simulink model "HvacCtrlrOsaAndRecircFrnt"  (R2022b)
settings: Solver=FixedStepDiscrete  StartTime=0.0  StopTime=10.0  FixedStep=0.2  RelTol=1e-3
settings Model: Creator=blue.ge  LastModifiedBy=blue.ge
settings DataIOCC: SaveFormat=StructureWithTime
settings OptimizationCC: DefaultParameterBehavior=Inlined  EnhancedBackFolding=on  PassReuseOutputArgsAs=Individual arguments  ZeroExternalMemoryAtStartup=off  ZeroInternalMemoryAtStartup=off  OptimizeBlockOrder=speed  DifferentSizesBufferReuse=on  OptimizationCustomize=off  EfficientTunableParamExpr=on
settings DebuggingCC: ParameterTunabilityLossMsg=error
settings ModelReferenceCC: ModelReferenceNumInstancesAllowed=Single
settings SFSimCC: MATLABDynamicMemAlloc=off
settings RTWCC: Description=AUTOSAR  SystemTargetFile=autosar.tlc  GenCodeOnly=on  TemplateMakefile=ert_default_tmf
settings CodeAppCC: ForceParamTrailComments=on  IgnoreCustomStorageClasses=off  OperatorAnnotations=on  SimulinkDataObjDesc=on  SFDataObjDesc=on  InsertBlockDesc=on
settings STFCustomTargetCC: TargetLangStandard=C99 (ISO)  MultiwordLength=256  ParMdlRefBuildCompliant=on  CompOptLevelCompliant=on  IncludeMdlTerminateFcn=off  CombineOutputUpdateFcns=on  SuppressErrorStatus=on  ERTCustomFileBanners=on  SupportNonFinite=off  SupportComplex=off  SupportContinuousTime=off  SupportNonInlinedSFcns=off  RemoveResetFunc=on  MATLABClassNameForMDSCustomization=Simulink.SoftwareTarget.ERTCustomization  AutosarCompliant=on  UseToolchainInfoCompliant=on  LookupTableObjectStructAxisOrder=2,1,3,4,...  LUTObjectStructOrderEvenSpacing=Size,Table,Breakpoints  SystemTargetFile=autosar.tlc
settings ERTTargetCC: TargetLangStandard=C99 (ISO)  ConcurrentExecutionCompliant=off  IncludeMdlTerminateFcn=off  SuppressErrorStatus=on  SupportNonFinite=off  SupportComplex=off  ModelStepFunctionPrototypeControlCompliant=off  CPPClassGenCompliant=off  AutosarCompliant=on  LookupTableObjectStructAxisOrder=2,1,3,4,...  LUTObjectStructOrderEvenSpacing=Size,Table,Breakpoints  InlinedParameterPlacement=NonHierarchical  ERTCustomFileTemplate=example_file_process.tlc

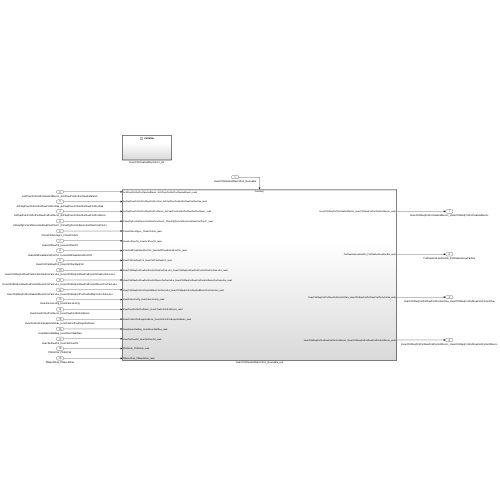
[thumbnail: Simulink's stored preview of the top-level diagram]
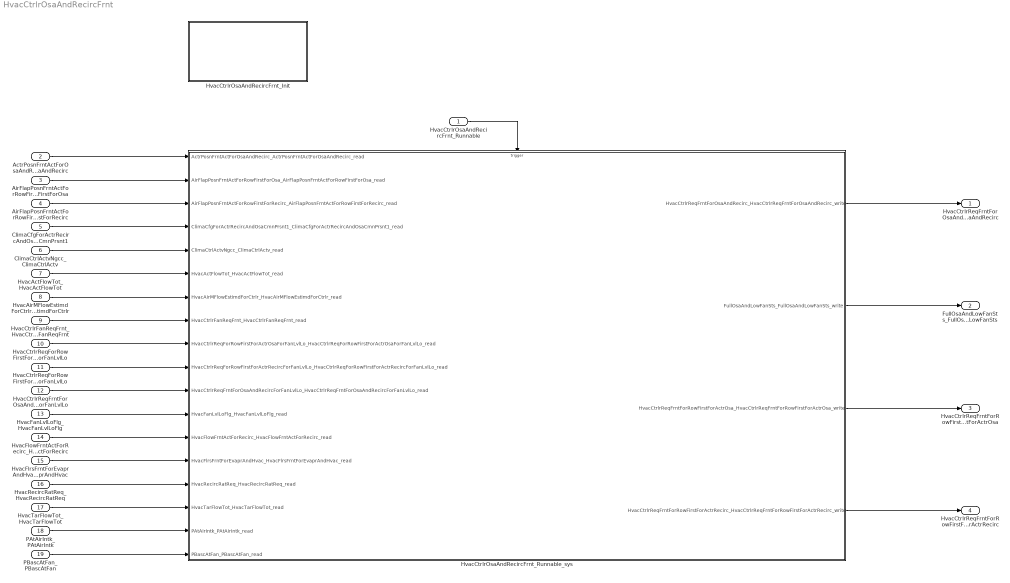
== system HvacCtrlrOsaAndRecircFrnt ==
  HvacCtrlrOsaAndRecircFrnt_Runnable : Inport  SampleTime=0.2  OutputFunctionCall=on
  ActrPosnFrntActForOsaAndRecirc_ActrPosnFrntActForOsaAndRecirc : Inport  Port=2  OutMin=0  OutMax=100  OutDataTypeStr=Perc9  PortDimensions=1
  AirFlapPosnFrntActForRowFirstForOsa_AirFlapPosnFrntActForRowFirstForOsa : Inport  Port=3  OutMin=0  OutMax=100  OutDataTypeStr=Perc9  PortDimensions=1
  AirFlapPosnFrntActForRowFirstForRecirc_AirFlapPosnFrntActForRowFirstForRecirc : Inport  Port=4  OutMin=0  OutMax=100  OutDataTypeStr=Perc9  PortDimensions=1
  ClimaCfgForActrRecircAndOsaCmnPrsnt1_ClimaCfgForActrRecircAndOsaCmnPrsnt1 : Inport  Port=5  OutDataTypeStr=Enum: ClimaCfgForActrRecircAndOsaCmnPrsnt1  PortDimensions=1
  ClimaCtrlActvNgcc_ClimaCtrlActv : Inport  Port=6  OutDataTypeStr=Boolean  PortDimensions=1
  HvacActFlowTot_HvacActFlowTot : Inport  Port=7  OutDataTypeStr=AirFlow30  PortDimensions=1
  HvacAirMFlowEstimdForCtrlr_HvacAirMFlowEstimdForCtrlr : Inport  Port=8  OutDataTypeStr=AirFlow30  PortDimensions=1
  HvacCtrlrFanReqFrnt_HvacCtrlrFanReqFrnt : Inport  Port=9  OutDataTypeStr=FanSpd1  PortDimensions=1
  HvacCtrlrReqForRowFirstForActrOsaForFanLvlLo_HvacCtrlrReqForRowFirstForActrOsaForFanLvlLo : Inport  Port=10  OutDataTypeStr=Perc30  PortDimensions=1
  HvacCtrlrReqForRowFirstForActrRecircForFanLvlLo_HvacCtrlrReqForRowFirstForActrRecircForFanLvlLo : Inport  Port=11  OutDataTypeStr=Perc30  PortDimensions=1
  HvacCtrlrReqFrntForOsaAndRecircForFanLvlLo_HvacCtrlrReqFrntForOsaAndRecircForFanLvlLo : Inport  Port=12  OutDataTypeStr=Perc30  PortDimensions=1
  HvacFanLvlLoFlg_HvacFanLvlLoFlg : Inport  Port=13  OutDataTypeStr=Boolean  PortDimensions=1
  HvacFlowFrntActForRecirc_HvacFlowFrntActForRecirc : Inport  Port=14  OutDataTypeStr=AirFlow30  PortDimensions=1
  HvacFlrsFrntForEvaprAndHvac_HvacFlrsFrntForEvaprAndHvac : Inport  Port=15  OutDataTypeStr=Flrs30  PortDimensions=1
  HvacRecircRatReq_HvacRecircRatReq : Inport  Port=16  OutDataTypeStr=Perc30  PortDimensions=1
  HvacTarFlowTot_HvacTarFlowTot : Inport  Port=17  OutDataTypeStr=AirFlow30  PortDimensions=1
  PAtAirIntk_PAtAirIntk : Inport  Port=18  OutDataTypeStr=AirP31  PortDimensions=1
  PBascAtFan_PBascAtFan : Inport  Port=19  OutDataTypeStr=AirP31  PortDimensions=1
  HvacCtrlrOsaAndRecircFrnt_Init : Subsystem
  HvacCtrlrOsaAndRecircFrnt_Runnable_sys : Subsystem
  HvacCtrlrReqFrntForOsaAndRecirc_HvacCtrlrReqFrntForOsaAndRecirc : Outport  OutDataTypeStr=Perc30  PortDimensions=1
  FullOsaAndLowFanSts_FullOsaAndLowFanSts : Outport  Port=2  OutDataTypeStr=Boolean  PortDimensions=1
  HvacCtrlrReqFrntForRowFirstForActrOsa_HvacCtrlrReqFrntForRowFirstForActrOsa : Outport  Port=3  OutDataTypeStr=Perc30  PortDimensions=1
  HvacCtrlrReqFrntForRowFirstForActrRecirc_HvacCtrlrReqFrntForRowFirstForActrRecirc : Outport  Port=4  OutDataTypeStr=Perc30  PortDimensions=1
  wires:
  ActrPosnFrntActForOsaAndRecirc_ActrPosnFrntActForOsaAndRecirc/1 -> HvacCtrlrOsaAndRecircFrnt_Runnable_sys/1(ActrPosnFrntActForOsaAndRecirc_ActrPosnFrntActForOsaAndRecirc_read)
  AirFlapPosnFrntActForRowFirstForOsa_AirFlapPosnFrntActForRowFirstForOsa/1 -> HvacCtrlrOsaAndRecircFrnt_Runnable_sys/2(AirFlapPosnFrntActForRowFirstForOsa_AirFlapPosnFrntActForRowFirstForOsa_read)
  AirFlapPosnFrntActForRowFirstForRecirc_AirFlapPosnFrntActForRowFirstForRecirc/1 -> HvacCtrlrOsaAndRecircFrnt_Runnable_sys/3(AirFlapPosnFrntActForRowFirstForRecirc_AirFlapPosnFrntActForRowFirstForRecirc_read)
  ClimaCfgForActrRecircAndOsaCmnPrsnt1_ClimaCfgForActrRecircAndOsaCmnPrsnt1/1 -> HvacCtrlrOsaAndRecircFrnt_Runnable_sys/4(ClimaCfgForActrRecircAndOsaCmnPrsnt1_ClimaCfgForActrRecircAndOsaCmnPrsnt1_read)
  ClimaCtrlActvNgcc_ClimaCtrlActv/1 -> HvacCtrlrOsaAndRecircFrnt_Runnable_sys/5(ClimaCtrlActvNgcc_ClimaCtrlActv_read)
  HvacActFlowTot_HvacActFlowTot/1 -> HvacCtrlrOsaAndRecircFrnt_Runnable_sys/6(HvacActFlowTot_HvacActFlowTot_read)
  HvacAirMFlowEstimdForCtrlr_HvacAirMFlowEstimdForCtrlr/1 -> HvacCtrlrOsaAndRecircFrnt_Runnable_sys/7(HvacAirMFlowEstimdForCtrlr_HvacAirMFlowEstimdForCtrlr_read)
  HvacCtrlrFanReqFrnt_HvacCtrlrFanReqFrnt/1 -> HvacCtrlrOsaAndRecircFrnt_Runnable_sys/8(HvacCtrlrFanReqFrnt_HvacCtrlrFanReqFrnt_read)
  HvacCtrlrReqForRowFirstForActrOsaForFanLvlLo_HvacCtrlrReqForRowFirstForActrOsaForFanLvlLo/1 -> HvacCtrlrOsaAndRecircFrnt_Runnable_sys/9(HvacCtrlrReqForRowFirstForActrOsaForFanLvlLo_HvacCtrlrReqForRowFirstForActrOsaForFanLvlLo_read)
  HvacCtrlrReqForRowFirstForActrRecircForFanLvlLo_HvacCtrlrReqForRowFirstForActrRecircForFanLvlLo/1 -> HvacCtrlrOsaAndRecircFrnt_Runnable_sys/10(HvacCtrlrReqForRowFirstForActrRecircForFanLvlLo_HvacCtrlrReqForRowFirstForActrRecircForFanLvlLo_read)
  HvacCtrlrReqFrntForOsaAndRecircForFanLvlLo_HvacCtrlrReqFrntForOsaAndRecircForFanLvlLo/1 -> HvacCtrlrOsaAndRecircFrnt_Runnable_sys/11(HvacCtrlrReqFrntForOsaAndRecircForFanLvlLo_HvacCtrlrReqFrntForOsaAndRecircForFanLvlLo_read)
  HvacFanLvlLoFlg_HvacFanLvlLoFlg/1 -> HvacCtrlrOsaAndRecircFrnt_Runnable_sys/12(HvacFanLvlLoFlg_HvacFanLvlLoFlg_read)
  HvacFlowFrntActForRecirc_HvacFlowFrntActForRecirc/1 -> HvacCtrlrOsaAndRecircFrnt_Runnable_sys/13(HvacFlowFrntActForRecirc_HvacFlowFrntActForRecirc_read)
  HvacFlrsFrntForEvaprAndHvac_HvacFlrsFrntForEvaprAndHvac/1 -> HvacCtrlrOsaAndRecircFrnt_Runnable_sys/14(HvacFlrsFrntForEvaprAndHvac_HvacFlrsFrntForEvaprAndHvac_read)
  HvacRecircRatReq_HvacRecircRatReq/1 -> HvacCtrlrOsaAndRecircFrnt_Runnable_sys/15(HvacRecircRatReq_HvacRecircRatReq_read)
  HvacTarFlowTot_HvacTarFlowTot/1 -> HvacCtrlrOsaAndRecircFrnt_Runnable_sys/16(HvacTarFlowTot_HvacTarFlowTot_read)
  PAtAirIntk_PAtAirIntk/1 -> HvacCtrlrOsaAndRecircFrnt_Runnable_sys/17(PAtAirIntk_PAtAirIntk_read)
  PBascAtFan_PBascAtFan/1 -> HvacCtrlrOsaAndRecircFrnt_Runnable_sys/18(PBascAtFan_PBascAtFan_read)
  HvacCtrlrOsaAndRecircFrnt_Runnable_sys/1(HvacCtrlrReqFrntForOsaAndRecirc_HvacCtrlrReqFrntForOsaAndRecirc_write) -> HvacCtrlrReqFrntForOsaAndRecirc_HvacCtrlrReqFrntForOsaAndRecirc/1
  HvacCtrlrOsaAndRecircFrnt_Runnable_sys/2(FullOsaAndLowFanSts_FullOsaAndLowFanSts_write) -> FullOsaAndLowFanSts_FullOsaAndLowFanSts/1
  HvacCtrlrOsaAndRecircFrnt_Runnable_sys/3(HvacCtrlrReqFrntForRowFirstForActrOsa_HvacCtrlrReqFrntForRowFirstForActrOsa_write) -> HvacCtrlrReqFrntForRowFirstForActrOsa_HvacCtrlrReqFrntForRowFirstForActrOsa/1
  HvacCtrlrOsaAndRecircFrnt_Runnable_sys/4(HvacCtrlrReqFrntForRowFirstForActrRecirc_HvacCtrlrReqFrntForRowFirstForActrRecirc_write) -> HvacCtrlrReqFrntForRowFirstForActrRecirc_HvacCtrlrReqFrntForRowFirstForActrRecirc/1
  HvacCtrlrOsaAndRecircFrnt_Runnable/1 -> HvacCtrlrOsaAndRecircFrnt_Runnable_sys/Trigger

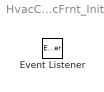
== system HvacCtrlrOsaAndRecircFrnt/HvacCtrlrOsaAndRecircFrnt_Init ==
  Event Listener : EventListener  EventName=reset

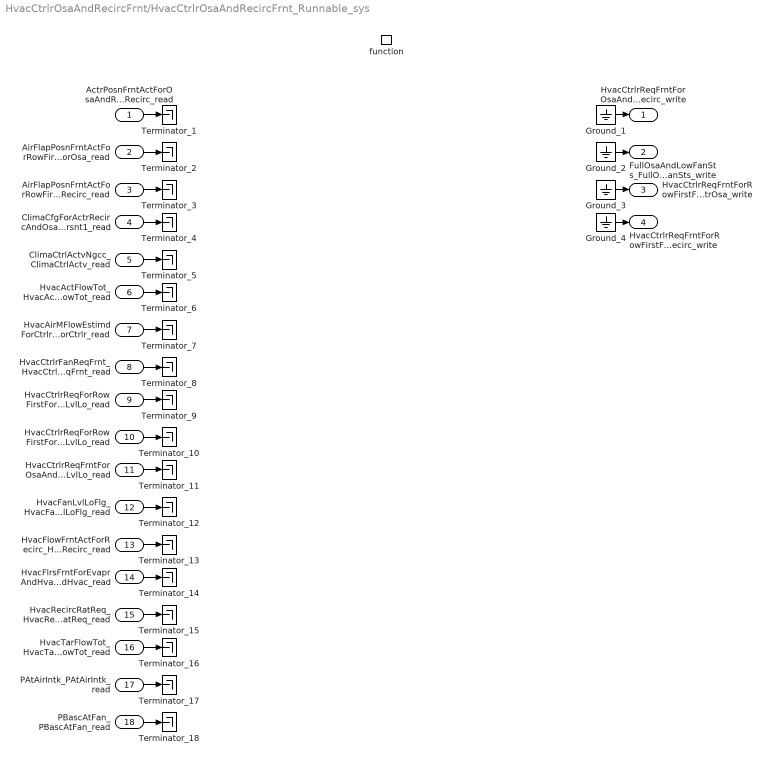
== system HvacCtrlrOsaAndRecircFrnt/HvacCtrlrOsaAndRecircFrnt_Runnable_sys ==
  ActrPosnFrntActForOsaAndRecirc_ActrPosnFrntActForOsaAndRecirc_read : Inport
  AirFlapPosnFrntActForRowFirstForOsa_AirFlapPosnFrntActForRowFirstForOsa_read : Inport  Port=2
  AirFlapPosnFrntActForRowFirstForRecirc_AirFlapPosnFrntActForRowFirstForRecirc_read : Inport  Port=3
  ClimaCfgForActrRecircAndOsaCmnPrsnt1_ClimaCfgForActrRecircAndOsaCmnPrsnt1_read : Inport  Port=4
  ClimaCtrlActvNgcc_ClimaCtrlActv_read : Inport  Port=5
  HvacActFlowTot_HvacActFlowTot_read : Inport  Port=6
  HvacAirMFlowEstimdForCtrlr_HvacAirMFlowEstimdForCtrlr_read : Inport  Port=7
  HvacCtrlrFanReqFrnt_HvacCtrlrFanReqFrnt_read : Inport  Port=8
  HvacCtrlrReqForRowFirstForActrOsaForFanLvlLo_HvacCtrlrReqForRowFirstForActrOsaForFanLvlLo_read : Inport  Port=9
  HvacCtrlrReqForRowFirstForActrRecircForFanLvlLo_HvacCtrlrReqForRowFirstForActrRecircForFanLvlLo_read : Inport  Port=10
  HvacCtrlrReqFrntForOsaAndRecircForFanLvlLo_HvacCtrlrReqFrntForOsaAndRecircForFanLvlLo_read : Inport  Port=11
  HvacFanLvlLoFlg_HvacFanLvlLoFlg_read : Inport  Port=12
  HvacFlowFrntActForRecirc_HvacFlowFrntActForRecirc_read : Inport  Port=13
  HvacFlrsFrntForEvaprAndHvac_HvacFlrsFrntForEvaprAndHvac_read : Inport  Port=14
  HvacRecircRatReq_HvacRecircRatReq_read : Inport  Port=15
  HvacTarFlowTot_HvacTarFlowTot_read : Inport  Port=16
  PAtAirIntk_PAtAirIntk_read : Inport  Port=17
  PBascAtFan_PBascAtFan_read : Inport  Port=18
  function : TriggerPort  TriggerType=function-call  VariantControl=(inherit)  SampleTimeType=periodic  SampleTime=-1
  Ground_{1..4} (4 alike) : Ground
  Terminator_{1..18} (18 alike) : Terminator
  HvacCtrlrReqFrntForOsaAndRecirc_HvacCtrlrReqFrntForOsaAndRecirc_write : Outport
      settings: EnsureOutportIsVirtual=on
  FullOsaAndLowFanSts_FullOsaAndLowFanSts_write : Outport  Port=2
      settings: EnsureOutportIsVirtual=on
  HvacCtrlrReqFrntForRowFirstForActrOsa_HvacCtrlrReqFrntForRowFirstForActrOsa_write : Outport  Port=3
      settings: EnsureOutportIsVirtual=on
  HvacCtrlrReqFrntForRowFirstForActrRecirc_HvacCtrlrReqFrntForRowFirstForActrRecirc_write : Outport  Port=4
      settings: EnsureOutportIsVirtual=on
  wires:
  ActrPosnFrntActForOsaAndRecirc_ActrPosnFrntActForOsaAndRecirc_read/1 -> Terminator_1/1
  AirFlapPosnFrntActForRowFirstForOsa_AirFlapPosnFrntActForRowFirstForOsa_read/1 -> Terminator_2/1
  AirFlapPosnFrntActForRowFirstForRecirc_AirFlapPosnFrntActForRowFirstForRecirc_read/1 -> Terminator_3/1
  ClimaCfgForActrRecircAndOsaCmnPrsnt1_ClimaCfgForActrRecircAndOsaCmnPrsnt1_read/1 -> Terminator_4/1
  ClimaCtrlActvNgcc_ClimaCtrlActv_read/1 -> Terminator_5/1
  HvacActFlowTot_HvacActFlowTot_read/1 -> Terminator_6/1
  HvacAirMFlowEstimdForCtrlr_HvacAirMFlowEstimdForCtrlr_read/1 -> Terminator_7/1
  HvacCtrlrFanReqFrnt_HvacCtrlrFanReqFrnt_read/1 -> Terminator_8/1
  HvacCtrlrReqForRowFirstForActrOsaForFanLvlLo_HvacCtrlrReqForRowFirstForActrOsaForFanLvlLo_read/1 -> Terminator_9/1
  HvacCtrlrReqForRowFirstForActrRecircForFanLvlLo_HvacCtrlrReqForRowFirstForActrRecircForFanLvlLo_read/1 -> Terminator_10/1
  HvacCtrlrReqFrntForOsaAndRecircForFanLvlLo_HvacCtrlrReqFrntForOsaAndRecircForFanLvlLo_read/1 -> Terminator_11/1
  HvacFanLvlLoFlg_HvacFanLvlLoFlg_read/1 -> Terminator_12/1
  HvacFlowFrntActForRecirc_HvacFlowFrntActForRecirc_read/1 -> Terminator_13/1
  HvacFlrsFrntForEvaprAndHvac_HvacFlrsFrntForEvaprAndHvac_read/1 -> Terminator_14/1
  HvacRecircRatReq_HvacRecircRatReq_read/1 -> Terminator_15/1
  HvacTarFlowTot_HvacTarFlowTot_read/1 -> Terminator_16/1
  PAtAirIntk_PAtAirIntk_read/1 -> Terminator_17/1
  PBascAtFan_PBascAtFan_read/1 -> Terminator_18/1
  Ground_1/1 -> HvacCtrlrReqFrntForOsaAndRecirc_HvacCtrlrReqFrntForOsaAndRecirc_write/1
  Ground_2/1 -> FullOsaAndLowFanSts_FullOsaAndLowFanSts_write/1
  Ground_3/1 -> HvacCtrlrReqFrntForRowFirstForActrOsa_HvacCtrlrReqFrntForRowFirstForActrOsa_write/1
  Ground_4/1 -> HvacCtrlrReqFrntForRowFirstForActrRecirc_HvacCtrlrReqFrntForRowFirstForActrRecirc_write/1
